simulink model "HvacCtrlrAirMFlowFrnt"  (R2022b)
settings: Solver=FixedStepDiscrete  StartTime=0.0  StopTime=10.0  FixedStep=0.2  RelTol=1e-3
(part 1 of 2)
settings Model: Creator=blue.ge  LastModifiedBy=blue.ge
settings DataIOCC: SaveFormat=StructureWithTime
settings OptimizationCC: DefaultParameterBehavior=Inlined  EnhancedBackFolding=on  PassReuseOutputArgsAs=Individual arguments  ZeroExternalMemoryAtStartup=off  ZeroInternalMemoryAtStartup=off  OptimizeBlockOrder=speed  DifferentSizesBufferReuse=on  OptimizationCustomize=off  EfficientTunableParamExpr=on
settings DebuggingCC: ParameterTunabilityLossMsg=error
settings ModelReferenceCC: ModelReferenceNumInstancesAllowed=Single
settings SFSimCC: MATLABDynamicMemAlloc=off
settings RTWCC: Description=AUTOSAR  SystemTargetFile=autosar.tlc  GenCodeOnly=on  TemplateMakefile=ert_default_tmf
settings CodeAppCC: ForceParamTrailComments=on  IgnoreCustomStorageClasses=off  OperatorAnnotations=on  SimulinkDataObjDesc=on  SFDataObjDesc=on  InsertBlockDesc=on
settings STFCustomTargetCC: TargetLangStandard=C99 (ISO)  MultiwordLength=256  ParMdlRefBuildCompliant=on  CompOptLevelCompliant=on  IncludeMdlTerminateFcn=off  CombineOutputUpdateFcns=on  SuppressErrorStatus=on  ERTCustomFileBanners=on  SupportNonFinite=off  SupportComplex=off  SupportContinuousTime=off  SupportNonInlinedSFcns=off  RemoveResetFunc=on  MATLABClassNameForMDSCustomization=Simulink.SoftwareTarget.ERTCustomization  AutosarCompliant=on  UseToolchainInfoCompliant=on  LookupTableObjectStructAxisOrder=2,1,3,4,...  LUTObjectStructOrderEvenSpacing=Size,Table,Breakpoints  SystemTargetFile=autosar.tlc
settings ERTTargetCC: TargetLangStandard=C99 (ISO)  ConcurrentExecutionCompliant=off  IncludeMdlTerminateFcn=off  SuppressErrorStatus=on  SupportNonFinite=off  SupportComplex=off  ModelStepFunctionPrototypeControlCompliant=off  CPPClassGenCompliant=off  AutosarCompliant=on  LookupTableObjectStructAxisOrder=2,1,3,4,...  LUTObjectStructOrderEvenSpacing=Size,Table,Breakpoints  InlinedParameterPlacement=NonHierarchical  ERTCustomFileTemplate=example_file_process.tlc

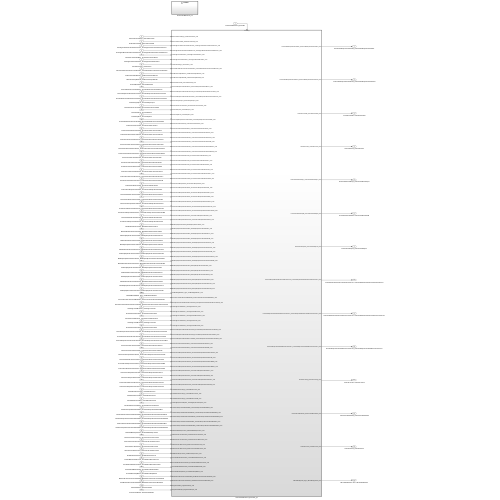
[thumbnail: Simulink's stored preview of the top-level diagram]
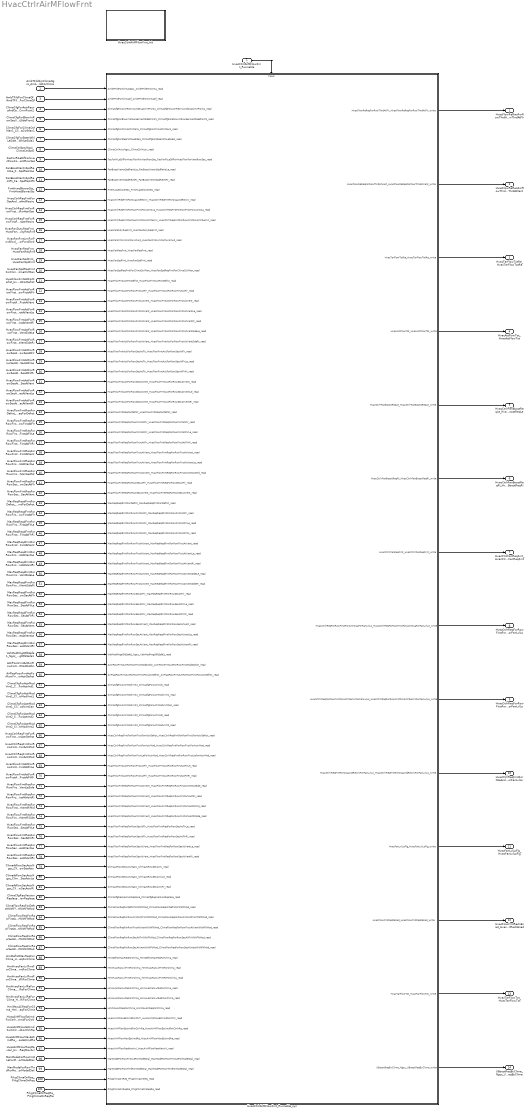
== system HvacCtrlrAirMFlowFrnt ==
  HvacCtrlrAirMFlowFrnt_Runnable : Inport  SampleTime=0.2  OutputFunctionCall=on
  AmbTFildForClimaNgcc_AmbTFildForClima : Inport  Port=2  OutDataTypeStr=T30  PortDimensions=1
  AmbTFildForClimaQf_AmbTFildForClimaQf : Inport  Port=3  OutDataTypeStr=Enum: GenQf1  PortDimensions=1
  ClimaCfgForActrRecircAndOsaCmnPrsnt1_ClimaCfgForActrRecircAndOsaCmnPrsnt1 : Inport  Port=4  OutDataTypeStr=Enum: ClimaCfgForActrRecircAndOsaCmnPrsnt1  PortDimensions=1
  ClimaCfgForBlowrInRowSecVentSidePrsnt1_ClimaCfgForBlowrInRowSecVentSidePrsnt1 : Inport  Port=5  OutDataTypeStr=Boolean  PortDimensions=1
  ClimaCfgForClimaCtrlMan1_ClimaCfgForClimaCtrlMan1 : Inport  Port=6  OutDataTypeStr=Boolean  PortDimensions=1
  ClimaCfgForSteerWhlLeSide1_ClimaCfgForSteerWhlLeSide1 : Inport  Port=7  OutDataTypeStr=Boolean  PortDimensions=1
  ClimaCtrlActvNgcc_ClimaCtrlActv : Inport  Port=8  OutDataTypeStr=Boolean  PortDimensions=1
  FacForRiLeDifForHvacFlowForVentRowSec_FacForRiLeDifForHvacFlowForVentRowSec : Inport  Port=9  OutDataTypeStr=Fac30  PortDimensions=1
  FanBoostVentnSpdReIntLe_FanBoostVentnSpdReIntLe : Inport  Port=10  OutDataTypeStr=HvacFanSpd  PortDimensions=1
  FanBoostVentnSpdReIntRi_FanBoostVentnSpdReIntRi : Inport  Port=11  OutDataTypeStr=HvacFanSpd  PortDimensions=1
  FrntHvacBlowerSts_FrntHvacBlowerSts : Inport  Port=12  OutDataTypeStr=Enum: HvacFanSts  PortDimensions=1
  HvacCtrlrReqFrntForOsaAndRecirc_HvacCtrlrReqFrntForOsaAndRecirc : Inport  Port=13  OutDataTypeStr=Perc30  PortDimensions=1
  HvacCtrlrReqFrntForRowFirstForActrOsa_HvacCtrlrReqFrntForRowFirstForActrOsa : Inport  Port=14  OutDataTypeStr=Perc30  PortDimensions=1
  HvacCtrlrReqFrntForRowFirstForActrRecirc_HvacCtrlrReqFrntForRowFirstForActrRecirc : Inport  Port=15  OutDataTypeStr=Perc30  PortDimensions=1
  HvacFanDutyReqFrnt_HvacFanDutyReqFrnt : Inport  Port=16  OutMin=0  OutMax=100  OutDataTypeStr=Perc9  PortDimensions=1
  HvacFanFrntLimForPwrAllwd_HvacFanFrntLimForPwrAllwd : Inport  Port=17  OutDataTypeStr=Enum: HvacFanFrntLimForPwrAllwd  PortDimensions=1
  HvacFanReqFrnt_HvacFanReqFrnt : Inport  Port=18  OutDataTypeStr=HvacFanSpd  PortDimensions=1
  HvacFanSpdFrnt_HvacFanSpdFrnt : Inport  Port=19  OutDataTypeStr=HvacFanSpd  PortDimensions=1
  HvacFanSpdReqFrntForClimaCtrlMan_HvacFanSpdReqFrntForClimaCtrlMan : Inport  Port=20  OutDataTypeStr=FanSpd1  PortDimensions=1
  HvacFlowFrntActForDefrst_HvacFlowFrntActForDefrst : Inport  Port=21  OutDataTypeStr=AirFlow30  PortDimensions=1
  HvacFlowFrntActForRowFirstAtFlr_HvacFlowFrntActForRowFirstAtFlr : Inport  Port=22  OutDataTypeStr=AirFlow30  PortDimensions=1
  HvacFlowFrntActForRowFirstAtVent_HvacFlowFrntActForRowFirstAtVent : Inport  Port=23  OutDataTypeStr=AirFlow30  PortDimensions=1
  HvacFlowFrntActForRowFirstAtVent_HvacFlowFrntActForRowFirstAtVentLe : Inport  Port=24  OutDataTypeStr=AirFlow30  PortDimensions=1
  HvacFlowFrntActForRowFirstAtVent_HvacFlowFrntActForRowFirstAtVentRi : Inport  Port=25  OutDataTypeStr=AirFlow30  PortDimensions=1
  HvacFlowFrntActForRowFirstAtVent_HvacFlowFrntActForRowFirstAtVentSideLe : Inport  Port=26  OutDataTypeStr=AirFlow30  PortDimensions=1
  HvacFlowFrntActForRowFirstAtVent_HvacFlowFrntActForRowFirstAtVentSideRi : Inport  Port=27  OutDataTypeStr=AirFlow30  PortDimensions=1
  HvacFlowFrntActForRowSecAtFlr_HvacFlowFrntActForRowSecAtFlr : Inport  Port=28  OutDataTypeStr=AirFlow30  PortDimensions=1
  HvacFlowFrntActForRowSecAtFlr_HvacFlowFrntActForRowSecAtFlrLe : Inport  Port=29  OutDataTypeStr=AirFlow30  PortDimensions=1
  HvacFlowFrntActForRowSecAtFlr_HvacFlowFrntActForRowSecAtFlrRi : Inport  Port=30  OutDataTypeStr=AirFlow30  PortDimensions=1
  HvacFlowFrntActForRowSecAtVent_HvacFlowFrntActForRowSecAtVent : Inport  Port=31  OutDataTypeStr=AirFlow30  PortDimensions=1
  HvacFlowFrntActForRowSecAtVent_HvacFlowFrntActForRowSecAtVentLe : Inport  Port=32  OutDataTypeStr=AirFlow30  PortDimensions=1
  HvacFlowFrntActForRowSecAtVent_HvacFlowFrntActForRowSecAtVentRi : Inport  Port=33  OutDataTypeStr=AirFlow30  PortDimensions=1
  HvacFlowFrntReqForDefrst_HvacFlowFrntReqForDefrst : Inport  Port=34  OutDataTypeStr=AirFlow30  PortDimensions=1
  HvacFlowFrntReqForRowFirstAtFlr_HvacFlowFrntReqForRowFirstAtFlr : Inport  Port=35  OutDataTypeStr=AirFlow30  PortDimensions=1
  HvacFlowFrntReqForRowFirstAtFlr_HvacFlowFrntReqForRowFirstAtFlrLe : Inport  Port=36  OutDataTypeStr=AirFlow30  PortDimensions=1
  HvacFlowFrntReqForRowFirstAtFlr_HvacFlowFrntReqForRowFirstAtFlrRi : Inport  Port=37  OutDataTypeStr=AirFlow30  PortDimensions=1
  HvacFlowFrntReqForRowFirstAtVent_HvacFlowFrntReqForRowFirstAtVent : Inport  Port=38  OutDataTypeStr=AirFlow30  PortDimensions=1
  HvacFlowFrntReqForRowFirstAtVent_HvacFlowFrntReqForRowFirstAtVentLe : Inport  Port=39  OutDataTypeStr=AirFlow30  PortDimensions=1
  HvacFlowFrntReqForRowFirstAtVent_HvacFlowFrntReqForRowFirstAtVentLeMid : Inport  Port=40  OutDataTypeStr=AirFlow30  PortDimensions=1
  HvacFlowFrntReqForRowSecAtFlr_HvacFlowFrntReqForRowSecAtFlr : Inport  Port=41  OutDataTypeStr=AirFlow30  PortDimensions=1
  HvacFlowFrntReqForRowSecAtVent_HvacFlowFrntReqForRowSecAtVent : Inport  Port=42  OutDataTypeStr=AirFlow30  PortDimensions=1
  MaxReqReqdFrntForDefrst_MaxReqReqdFrntForDefrst : Inport  Port=43  OutDataTypeStr=Boolean  PortDimensions=1
  MaxReqReqdFrntForRowFirstAtFlr_MaxReqReqdFrntForRowFirstAtFlr : Inport  Port=44  OutDataTypeStr=Boolean  PortDimensions=1
  MaxReqReqdFrntForRowFirstAtFlr_MaxReqReqdFrntForRowFirstAtFlrLe : Inport  Port=45  OutDataTypeStr=Boolean  PortDimensions=1
  MaxReqReqdFrntForRowFirstAtFlr_MaxReqReqdFrntForRowFirstAtFlrRi : Inport  Port=46  OutDataTypeStr=Boolean  PortDimensions=1
  MaxReqReqdFrntForRowFirstAtVent_MaxReqReqdFrntForRowFirstAtVent : Inport  Port=47  OutDataTypeStr=Boolean  PortDimensions=1
  MaxReqReqdFrntForRowFirstAtVent_MaxReqReqdFrntForRowFirstAtVentLe : Inport  Port=48  OutDataTypeStr=Boolean  PortDimensions=1
  MaxReqReqdFrntForRowFirstAtVent_MaxReqReqdFrntForRowFirstAtVentRi : Inport  Port=49  OutDataTypeStr=Boolean  PortDimensions=1
  MaxReqReqdFrntForRowFirstAtVent_MaxReqReqdFrntForRowFirstAtVentSideLe : Inport  Port=50  OutDataTypeStr=Boolean  PortDimensions=1
  MaxReqReqdFrntForRowFirstAtVent_MaxReqReqdFrntForRowFirstAtVentSideRi : Inport  Port=51  OutDataTypeStr=Boolean  PortDimensions=1
  MaxReqReqdFrntForRowSecAtFlr_MaxReqReqdFrntForRowSecAtFlr : Inport  Port=52  OutDataTypeStr=Boolean  PortDimensions=1
  MaxReqReqdFrntForRowSecAtFlr_MaxReqReqdFrntForRowSecAtFlrLe : Inport  Port=53  OutDataTypeStr=Boolean  PortDimensions=1
  MaxReqReqdFrntForRowSecAtFlr_MaxReqReqdFrntForRowSecAtFlrRi : Inport  Port=54  OutDataTypeStr=Boolean  PortDimensions=1
  MaxReqReqdFrntForRowSecAtVent_MaxReqReqdFrntForRowSecAtVent : Inport  Port=55  OutDataTypeStr=Boolean  PortDimensions=1
  MaxReqReqdFrntForRowSecAtVent_MaxReqReqdFrntForRowSecAtVentLe : Inport  Port=56  OutDataTypeStr=Boolean  PortDimensions=1
  MaxReqReqdFrntForRowSecAtVent_MaxReqReqdFrntForRowSecAtVentRi : Inport  Port=57  OutDataTypeStr=Boolean  PortDimensions=1
  VehModMngtGlbSafe1_Ngcc_VehModMngtGlbSafe1 : Inport  Port=58  OutDataTypeStr=Bus: VehModMngtGlbSafe1  PortDimensions=1  BusOutputAsStruct=on
  ActrPosnFrntActForRowFirstModDistbn_ActrPosnFrntActForRowFirstModDistbn : Inport  Port=59  OutMin=0  OutMax=100  OutDataTypeStr=Perc9  PortDimensions=1
  AirFlapPosnFrntActForRowFirstForActrDefrst_AirFlapPosnFrntActForRowFirstForActrDefrst : Inport  Port=60  OutMin=0  OutMax=100  OutDataTypeStr=Perc9  PortDimensions=1
  ClimaCfgForActrModVrnt1_ClimaCfgForActrInd1 : Inport  Port=61  OutDataTypeStr=Enum: ClimaCfgForActrInd1  PortDimensions=1
  ClimaCfgForActrModVrnt1_ClimaCfgForActrModVrnt1 : Inport  Port=62  OutDataTypeStr=Enum: ClimaCfgForActrModVrnt1  PortDimensions=1
  ClimaCfgForActrModVrnt1_ClimaCfgForActrModVrntCen : Inport  Port=63  OutDataTypeStr=Enum: ClimaCfgForActrModVrntCen  PortDimensions=1
  ClimaCfgForActrModVrnt2_ClimaCfgForActrInd2 : Inport  Port=64  OutDataTypeStr=Enum: ClimaCfgForActrInd2  PortDimensions=1
  ClimaCfgForActrModVrnt2_ClimaCfgForActrModVrnt2 : Inport  Port=65  OutDataTypeStr=Enum: ClimaCfgForActrModVrnt2  PortDimensions=1
  HvacCtrlrReqFrntForRowFirstForActrDefrst_HvacCtrlrReqFrntForRowFirstForActrDefrst : Inport  Port=66  OutDataTypeStr=Perc30  PortDimensions=1
  HvacCtrlrReqFrntForRowFirstForActrMod_HvacCtrlrReqFrntForRowFirstForActrMod : Inport  Port=67  OutDataTypeStr=Perc30  PortDimensions=1
  HvacCtrlrReqFrntForRowFirstLeForActrMod_HvacCtrlrReqFrntForRowFirstLeForActrMod : Inport  Port=68  OutDataTypeStr=Perc30  PortDimensions=1
  HvacFlowFrntActForRowFirstAtFlr_HvacFlowFrntActForRowFirstAtFlrLe : Inport  Port=69  OutDataTypeStr=AirFlow30  PortDimensions=1
  HvacFlowFrntActForRowFirstAtFlr_HvacFlowFrntActForRowFirstAtFlrRi : Inport  Port=70  OutDataTypeStr=AirFlow30  PortDimensions=1
  HvacFlowFrntReqForRowFirstAtVent_HvacFlowFrntReqForRowFirstAtVentLeSide : Inport  Port=71  OutDataTypeStr=AirFlow30  PortDimensions=1
  HvacFlowFrntReqForRowFirstAtVent_HvacFlowFrntReqForRowFirstAtVentRi : Inport  Port=72  OutDataTypeStr=AirFlow30  PortDimensions=1
  HvacFlowFrntReqForRowFirstAtVent_HvacFlowFrntReqForRowFirstAtVentRiMid : Inport  Port=73  OutDataTypeStr=AirFlow30  PortDimensions=1
  HvacFlowFrntReqForRowFirstAtVent_HvacFlowFrntReqForRowFirstAtVentRiSide : Inport  Port=74  OutDataTypeStr=AirFlow30  PortDimensions=1
  HvacFlowFrntReqForRowSecAtFlr_HvacFlowFrntReqForRowSecAtFlrLe : Inport  Port=75  OutDataTypeStr=AirFlow30  PortDimensions=1
  HvacFlowFrntReqForRowSecAtFlr_HvacFlowFrntReqForRowSecAtFlrRi : Inport  Port=76  OutDataTypeStr=AirFlow30  PortDimensions=1
  HvacFlowFrntReqForRowSecAtVent_HvacFlowFrntReqForRowSecAtVentLe : Inport  Port=77  OutDataTypeStr=AirFlow30  PortDimensions=1
  HvacFlowFrntReqForRowSecAtVent_HvacFlowFrntReqForRowSecAtVentRi : Inport  Port=78  OutDataTypeStr=AirFlow30  PortDimensions=1
  ClimaAtRowSecActvNgcc_ClimaAtRowSecActv : Inport  Port=79  OutDataTypeStr=Boolean  PortDimensions=1
  ClimaAtRowSecActvNgcc_ClimaAtRowSecActvLe : Inport  Port=80  OutDataTypeStr=Boolean  PortDimensions=1
  ClimaAtRowSecActvNgcc_ClimaAtRowSecActvRi : Inport  Port=81  OutDataTypeStr=Boolean  PortDimensions=1
  ClimaCfgReqVersionReplace_ClimaCfgReqVersionReplace : Inport  Port=82  OutDataTypeStr=UInt8  PortDimensions=1
  ClimaFlowReqForDefrstWithFltMod_ClimaFlowReqForDefrstWithFltMod : Inport  Port=83  OutDataTypeStr=AirFlow30  PortDimensions=1
  ClimaFlowReqForRowFirstAtFlrWithFltMod_ClimaFlowReqForRowFirstAtFlrWithFltMod : Inport  Port=84  OutDataTypeStr=AirFlow30  PortDimensions=1
  ClimaFlowReqForRowFirstAtVentWithFltMod_ClimaFlowReqForRowFirstAtVentWithFltMod : Inport  Port=85  OutDataTypeStr=AirFlow30  PortDimensions=1
  ClimaFlowReqForRowSecAtFlrWithFltMod_ClimaFlowReqForRowSecAtFlrWithFltMod : Inport  Port=86  OutDataTypeStr=AirFlow30  PortDimensions=1
  ClimaFlowReqForRowSecAtVentWithFltMod_ClimaFlowReqForRowSecAtVentWithFltMod : Inport  Port=87  OutDataTypeStr=AirFlow30  PortDimensions=1
  HmiDefrstMaxReqForClima_HmiDefrstMaxReqForClima : Inport  Port=88  OutDataTypeStr=Enum: ActrReq  PortDimensions=1
  HmiHvacFanLvlFrntForClima_HmiHvacFanLvlFrntForClima : Inport  Port=89  OutDataTypeStr=Enum: HmiHvacFanLvl  PortDimensions=1
  HmiHvacFanLvlFrntForClima_HmiHvacFanLvlFrntRiForClima : Inport  Port=90  OutDataTypeStr=Enum: HmiHvacFanLvl  PortDimensions=1
  HmiHvacFanLvlReForClima_HmiHvacFanLvlReForClima : Inport  Port=91  OutDataTypeStr=Enum: HmiHvacFanLvl  PortDimensions=1
  HmiHvacFanLvlReForClima_HmiHvacFanLvlReRiForClima : Inport  Port=92  OutDataTypeStr=Enum: HmiHvacFanLvl  PortDimensions=1
  HmiMaxACReqForClima_HmiMaxACReqForClima : Inport  Port=93  OutDataTypeStr=Enum: OnOff1  PortDimensions=1
  HvacAirMFlowEstimdForCtrlr_HvacAirMFlowEstimdForCtrlr : Inport  Port=94  OutDataTypeStr=AirFlow30  PortDimensions=1
  HvacAirMFlowEstimdForCtrlrRe_HvacAirMFlowEstimdForCtrlrRe : Inport  Port=95  OutDataTypeStr=AirFlow30  PortDimensions=1
  HvacAirMFlowMaxEstimdRe_HvacAirMFlowMaxEstimdRe : Inport  Port=96  OutDataTypeStr=AirFlow30  PortDimensions=1
  HvacAirMFlowReqMaxAvl_HvacAirMFlowReqMaxAvl : Inport  Port=97  OutDataTypeStr=AirFlow30  PortDimensions=1
  ManModeForRowFirstLeForModelDial_ManModeForRowFirstLeForModelDial : Inport  Port=98  OutDataTypeStr=Enum: HmiCmptmtAirDistbnFrnt  PortDimensions=1
  ManModeForRowThrdForModelDial_ManModeForRowThrdForModelDial : Inport  Port=99  OutDataTypeStr=Enum: HmiCmptmtAirDistbnFrnt  PortDimensions=1
  PrkgClimaOnReq_PrkgClimaOnReq : Inport  Port=100  OutDataTypeStr=Boolean  PortDimensions=1
  PrkgClimaOnReqRe_PrkgClimaOnReqRe : Inport  Port=101  OutDataTypeStr=Enum: OnOff1  PortDimensions=1
  HvacCtrlrAirMFlowFrnt_Init : Subsystem
  HvacCtrlrAirMFlowFrnt_Runnable_sys : Subsystem
  HvacFlowReReqForRowThrdAtFlr_HvacFlowReReqForRowThrdAtFlr : Outport  OutDataTypeStr=AirFlow30  PortDimensions=1
  HvacFlowReReqForRowThrdAtVent_HvacFlowReReqForRowThrdAtVent : Outport  Port=2  OutDataTypeStr=AirFlow30  PortDimensions=1
  HvacTarFlowTotRe_HvacTarFlowTotRe : Outport  Port=3  OutDataTypeStr=AirFlow30  PortDimensions=1
  HvacActFlowTot_HvacActFlowTot : Outport  Port=4  OutDataTypeStr=AirFlow30  PortDimensions=1
  HvacCtrlrFanBoostReqLe_HvacCtrlrFanBoostReqLe : Outport  Port=5  OutDataTypeStr=Perc30  PortDimensions=1
  HvacCtrlrFanBoostReqRi_HvacCtrlrFanBoostReqRi : Outport  Port=6  OutDataTypeStr=Perc30  PortDimensions=1
  HvacCtrlrFanReqFrnt_HvacCtrlrFanReqFrnt : Outport  Port=7  OutDataTypeStr=FanSpd1  PortDimensions=1
  HvacCtrlrReqForRowFirstForActrOsaForFanLvlLo_HvacCtrlrReqForRowFirstForActrOsaForFanLvlLo : Outport  Port=8  OutDataTypeStr=Perc30  PortDimensions=1
  HvacCtrlrReqForRowFirstForActrRecircForFanLvlLo_HvacCtrlrReqForRowFirstForActrRecircForFanLvlLo : Outport  Port=9  OutDataTypeStr=Perc30  PortDimensions=1
  HvacCtrlrReqFrntForOsaAndRecircForFanLvlLo_HvacCtrlrReqFrntForOsaAndRecircForFanLvlLo : Outport  Port=10  OutDataTypeStr=Perc30  PortDimensions=1
  HvacFanLvlLoFlg_HvacFanLvlLoFlg : Outport  Port=11  OutDataTypeStr=Boolean  PortDimensions=1
  HvacFlowFrntRednEnad_HvacFlowFrntRednEnad : Outport  Port=12  OutDataTypeStr=Boolean  PortDimensions=1
  HvacTarFlowTot_HvacTarFlowTot : Outport  Port=13  OutDataTypeStr=AirFlow30  PortDimensions=1
  UBoostReqByClima_Ngcc_UBoostReqByClima : Outport  Port=14  OutDataTypeStr=Enum: ReqSts1  PortDimensions=1
  wires:
  AmbTFildForClimaNgcc_AmbTFildForClima/1 -> HvacCtrlrAirMFlowFrnt_Runnable_sys/1(AmbTFildForClimaNgcc_AmbTFildForClima_read)
  AmbTFildForClimaQf_AmbTFildForClimaQf/1 -> HvacCtrlrAirMFlowFrnt_Runnable_sys/2(AmbTFildForClimaQf_AmbTFildForClimaQf_read)
  ClimaCfgForActrRecircAndOsaCmnPrsnt1_ClimaCfgForActrRecircAndOsaCmnPrsnt1/1 -> HvacCtrlrAirMFlowFrnt_Runnable_sys/3(ClimaCfgForActrRecircAndOsaCmnPrsnt1_ClimaCfgForActrRecircAndOsaCmnPrsnt1_read)
  ClimaCfgForBlowrInRowSecVentSidePrsnt1_ClimaCfgForBlowrInRowSecVentSidePrsnt1/1 -> HvacCtrlrAirMFlowFrnt_Runnable_sys/4(ClimaCfgForBlowrInRowSecVentSidePrsnt1_ClimaCfgForBlowrInRowSecVentSidePrsnt1_read)
  ClimaCfgForClimaCtrlMan1_ClimaCfgForClimaCtrlMan1/1 -> HvacCtrlrAirMFlowFrnt_Runnable_sys/5(ClimaCfgForClimaCtrlMan1_ClimaCfgForClimaCtrlMan1_read)
  ClimaCfgForSteerWhlLeSide1_ClimaCfgForSteerWhlLeSide1/1 -> HvacCtrlrAirMFlowFrnt_Runnable_sys/6(ClimaCfgForSteerWhlLeSide1_ClimaCfgForSteerWhlLeSide1_read)
  ClimaCtrlActvNgcc_ClimaCtrlActv/1 -> HvacCtrlrAirMFlowFrnt_Runnable_sys/7(ClimaCtrlActvNgcc_ClimaCtrlActv_read)
  FacForRiLeDifForHvacFlowForVentRowSec_FacForRiLeDifForHvacFlowForVentRowSec/1 -> HvacCtrlrAirMFlowFrnt_Runnable_sys/8(FacForRiLeDifForHvacFlowForVentRowSec_FacForRiLeDifForHvacFlowForVentRowSec_read)
  FanBoostVentnSpdReIntLe_FanBoostVentnSpdReIntLe/1 -> HvacCtrlrAirMFlowFrnt_Runnable_sys/9(FanBoostVentnSpdReIntLe_FanBoostVentnSpdReIntLe_read)
  FanBoostVentnSpdReIntRi_FanBoostVentnSpdReIntRi/1 -> HvacCtrlrAirMFlowFrnt_Runnable_sys/10(FanBoostVentnSpdReIntRi_FanBoostVentnSpdReIntRi_read)
  FrntHvacBlowerSts_FrntHvacBlowerSts/1 -> HvacCtrlrAirMFlowFrnt_Runnable_sys/11(FrntHvacBlowerSts_FrntHvacBlowerSts_read)
  HvacCtrlrReqFrntForOsaAndRecirc_HvacCtrlrReqFrntForOsaAndRecirc/1 -> HvacCtrlrAirMFlowFrnt_Runnable_sys/12(HvacCtrlrReqFrntForOsaAndRecirc_HvacCtrlrReqFrntForOsaAndRecirc_read)
  HvacCtrlrReqFrntForRowFirstForActrOsa_HvacCtrlrReqFrntForRowFirstForActrOsa/1 -> HvacCtrlrAirMFlowFrnt_Runnable_sys/13(HvacCtrlrReqFrntForRowFirstForActrOsa_HvacCtrlrReqFrntForRowFirstForActrOsa_read)
  HvacCtrlrReqFrntForRowFirstForActrRecirc_HvacCtrlrReqFrntForRowFirstForActrRecirc/1 -> HvacCtrlrAirMFlowFrnt_Runnable_sys/14(HvacCtrlrReqFrntForRowFirstForActrRecirc_HvacCtrlrReqFrntForRowFirstForActrRecirc_read)
  HvacFanDutyReqFrnt_HvacFanDutyReqFrnt/1 -> HvacCtrlrAirMFlowFrnt_Runnable_sys/15(HvacFanDutyReqFrnt_HvacFanDutyReqFrnt_read)
  HvacFanFrntLimForPwrAllwd_HvacFanFrntLimForPwrAllwd/1 -> HvacCtrlrAirMFlowFrnt_Runnable_sys/16(HvacFanFrntLimForPwrAllwd_HvacFanFrntLimForPwrAllwd_read)
  HvacFanReqFrnt_HvacFanReqFrnt/1 -> HvacCtrlrAirMFlowFrnt_Runnable_sys/17(HvacFanReqFrnt_HvacFanReqFrnt_read)
  HvacFanSpdFrnt_HvacFanSpdFrnt/1 -> HvacCtrlrAirMFlowFrnt_Runnable_sys/18(HvacFanSpdFrnt_HvacFanSpdFrnt_read)
  HvacFanSpdReqFrntForClimaCtrlMan_HvacFanSpdReqFrntForClimaCtrlMan/1 -> HvacCtrlrAirMFlowFrnt_Runnable_sys/19(HvacFanSpdReqFrntForClimaCtrlMan_HvacFanSpdReqFrntForClimaCtrlMan_read)
  HvacFlowFrntActForDefrst_HvacFlowFrntActForDefrst/1 -> HvacCtrlrAirMFlowFrnt_Runnable_sys/20(HvacFlowFrntActForDefrst_HvacFlowFrntActForDefrst_read)
  HvacFlowFrntActForRowFirstAtFlr_HvacFlowFrntActForRowFirstAtFlr/1 -> HvacCtrlrAirMFlowFrnt_Runnable_sys/21(HvacFlowFrntActForRowFirstAtFlr_HvacFlowFrntActForRowFirstAtFlr_read)
  HvacFlowFrntActForRowFirstAtVent_HvacFlowFrntActForRowFirstAtVent/1 -> HvacCtrlrAirMFlowFrnt_Runnable_sys/22(HvacFlowFrntActForRowFirstAtVent_HvacFlowFrntActForRowFirstAtVent_read)
  HvacFlowFrntActForRowFirstAtVent_HvacFlowFrntActForRowFirstAtVentLe/1 -> HvacCtrlrAirMFlowFrnt_Runnable_sys/23(HvacFlowFrntActForRowFirstAtVent_HvacFlowFrntActForRowFirstAtVentLe_read)
  HvacFlowFrntActForRowFirstAtVent_HvacFlowFrntActForRowFirstAtVentRi/1 -> HvacCtrlrAirMFlowFrnt_Runnable_sys/24(HvacFlowFrntActForRowFirstAtVent_HvacFlowFrntActForRowFirstAtVentRi_read)
  HvacFlowFrntActForRowFirstAtVent_HvacFlowFrntActForRowFirstAtVentSideLe/1 -> HvacCtrlrAirMFlowFrnt_Runnable_sys/25(HvacFlowFrntActForRowFirstAtVent_HvacFlowFrntActForRowFirstAtVentSideLe_read)
  HvacFlowFrntActForRowFirstAtVent_HvacFlowFrntActForRowFirstAtVentSideRi/1 -> HvacCtrlrAirMFlowFrnt_Runnable_sys/26(HvacFlowFrntActForRowFirstAtVent_HvacFlowFrntActForRowFirstAtVentSideRi_read)
  HvacFlowFrntActForRowSecAtFlr_HvacFlowFrntActForRowSecAtFlr/1 -> HvacCtrlrAirMFlowFrnt_Runnable_sys/27(HvacFlowFrntActForRowSecAtFlr_HvacFlowFrntActForRowSecAtFlr_read)
  HvacFlowFrntActForRowSecAtFlr_HvacFlowFrntActForRowSecAtFlrLe/1 -> HvacCtrlrAirMFlowFrnt_Runnable_sys/28(HvacFlowFrntActForRowSecAtFlr_HvacFlowFrntActForRowSecAtFlrLe_read)
  HvacFlowFrntActForRowSecAtFlr_HvacFlowFrntActForRowSecAtFlrRi/1 -> HvacCtrlrAirMFlowFrnt_Runnable_sys/29(HvacFlowFrntActForRowSecAtFlr_HvacFlowFrntActForRowSecAtFlrRi_read)
  HvacFlowFrntActForRowSecAtVent_HvacFlowFrntActForRowSecAtVent/1 -> HvacCtrlrAirMFlowFrnt_Runnable_sys/30(HvacFlowFrntActForRowSecAtVent_HvacFlowFrntActForRowSecAtVent_read)
  HvacFlowFrntActForRowSecAtVent_HvacFlowFrntActForRowSecAtVentLe/1 -> HvacCtrlrAirMFlowFrnt_Runnable_sys/31(HvacFlowFrntActForRowSecAtVent_HvacFlowFrntActForRowSecAtVentLe_read)
  HvacFlowFrntActForRowSecAtVent_HvacFlowFrntActForRowSecAtVentRi/1 -> HvacCtrlrAirMFlowFrnt_Runnable_sys/32(HvacFlowFrntActForRowSecAtVent_HvacFlowFrntActForRowSecAtVentRi_read)
  HvacFlowFrntReqForDefrst_HvacFlowFrntReqForDefrst/1 -> HvacCtrlrAirMFlowFrnt_Runnable_sys/33(HvacFlowFrntReqForDefrst_HvacFlowFrntReqForDefrst_read)
  HvacFlowFrntReqForRowFirstAtFlr_HvacFlowFrntReqForRowFirstAtFlr/1 -> HvacCtrlrAirMFlowFrnt_Runnable_sys/34(HvacFlowFrntReqForRowFirstAtFlr_HvacFlowFrntReqForRowFirstAtFlr_read)
  HvacFlowFrntReqForRowFirstAtFlr_HvacFlowFrntReqForRowFirstAtFlrLe/1 -> HvacCtrlrAirMFlowFrnt_Runnable_sys/35(HvacFlowFrntReqForRowFirstAtFlr_HvacFlowFrntReqForRowFirstAtFlrLe_read)
  HvacFlowFrntReqForRowFirstAtFlr_HvacFlowFrntReqForRowFirstAtFlrRi/1 -> HvacCtrlrAirMFlowFrnt_Runnable_sys/36(HvacFlowFrntReqForRowFirstAtFlr_HvacFlowFrntReqForRowFirstAtFlrRi_read)
  HvacFlowFrntReqForRowFirstAtVent_HvacFlowFrntReqForRowFirstAtVent/1 -> HvacCtrlrAirMFlowFrnt_Runnable_sys/37(HvacFlowFrntReqForRowFirstAtVent_HvacFlowFrntReqForRowFirstAtVent_read)
  HvacFlowFrntReqForRowFirstAtVent_HvacFlowFrntReqForRowFirstAtVentLe/1 -> HvacCtrlrAirMFlowFrnt_Runnable_sys/38(HvacFlowFrntReqForRowFirstAtVent_HvacFlowFrntReqForRowFirstAtVentLe_read)
  HvacFlowFrntReqForRowFirstAtVent_HvacFlowFrntReqForRowFirstAtVentLeMid/1 -> HvacCtrlrAirMFlowFrnt_Runnable_sys/39(HvacFlowFrntReqForRowFirstAtVent_HvacFlowFrntReqForRowFirstAtVentLeMid_read)
  HvacFlowFrntReqForRowSecAtFlr_HvacFlowFrntReqForRowSecAtFlr/1 -> HvacCtrlrAirMFlowFrnt_Runnable_sys/40(HvacFlowFrntReqForRowSecAtFlr_HvacFlowFrntReqForRowSecAtFlr_read)
  HvacFlowFrntReqForRowSecAtVent_HvacFlowFrntReqForRowSecAtVent/1 -> HvacCtrlrAirMFlowFrnt_Runnable_sys/41(HvacFlowFrntReqForRowSecAtVent_HvacFlowFrntReqForRowSecAtVent_read)
  MaxReqReqdFrntForDefrst_MaxReqReqdFrntForDefrst/1 -> HvacCtrlrAirMFlowFrnt_Runnable_sys/42(MaxReqReqdFrntForDefrst_MaxReqReqdFrntForDefrst_read)
  MaxReqReqdFrntForRowFirstAtFlr_MaxReqReqdFrntForRowFirstAtFlr/1 -> HvacCtrlrAirMFlowFrnt_Runnable_sys/43(MaxReqReqdFrntForRowFirstAtFlr_MaxReqReqdFrntForRowFirstAtFlr_read)
  MaxReqReqdFrntForRowFirstAtFlr_MaxReqReqdFrntForRowFirstAtFlrLe/1 -> HvacCtrlrAirMFlowFrnt_Runnable_sys/44(MaxReqReqdFrntForRowFirstAtFlr_MaxReqReqdFrntForRowFirstAtFlrLe_read)
  MaxReqReqdFrntForRowFirstAtFlr_MaxReqReqdFrntForRowFirstAtFlrRi/1 -> HvacCtrlrAirMFlowFrnt_Runnable_sys/45(MaxReqReqdFrntForRowFirstAtFlr_MaxReqReqdFrntForRowFirstAtFlrRi_read)
  MaxReqReqdFrntForRowFirstAtVent_MaxReqReqdFrntForRowFirstAtVent/1 -> HvacCtrlrAirMFlowFrnt_Runnable_sys/46(MaxReqReqdFrntForRowFirstAtVent_MaxReqReqdFrntForRowFirstAtVent_read)
  MaxReqReqdFrntForRowFirstAtVent_MaxReqReqdFrntForRowFirstAtVentLe/1 -> HvacCtrlrAirMFlowFrnt_Runnable_sys/47(MaxReqReqdFrntForRowFirstAtVent_MaxReqReqdFrntForRowFirstAtVentLe_read)
  MaxReqReqdFrntForRowFirstAtVent_MaxReqReqdFrntForRowFirstAtVentRi/1 -> HvacCtrlrAirMFlowFrnt_Runnable_sys/48(MaxReqReqdFrntForRowFirstAtVent_MaxReqReqdFrntForRowFirstAtVentRi_read)
  MaxReqReqdFrntForRowFirstAtVent_MaxReqReqdFrntForRowFirstAtVentSideLe/1 -> HvacCtrlrAirMFlowFrnt_Runnable_sys/49(MaxReqReqdFrntForRowFirstAtVent_MaxReqReqdFrntForRowFirstAtVentSideLe_read)
  MaxReqReqdFrntForRowFirstAtVent_MaxReqReqdFrntForRowFirstAtVentSideRi/1 -> HvacCtrlrAirMFlowFrnt_Runnable_sys/50(MaxReqReqdFrntForRowFirstAtVent_MaxReqReqdFrntForRowFirstAtVentSideRi_read)
  MaxReqReqdFrntForRowSecAtFlr_MaxReqReqdFrntForRowSecAtFlr/1 -> HvacCtrlrAirMFlowFrnt_Runnable_sys/51(MaxReqReqdFrntForRowSecAtFlr_MaxReqReqdFrntForRowSecAtFlr_read)
  MaxReqReqdFrntForRowSecAtFlr_MaxReqReqdFrntForRowSecAtFlrLe/1 -> HvacCtrlrAirMFlowFrnt_Runnable_sys/52(MaxReqReqdFrntForRowSecAtFlr_MaxReqReqdFrntForRowSecAtFlrLe_read)
  MaxReqReqdFrntForRowSecAtFlr_MaxReqReqdFrntForRowSecAtFlrRi/1 -> HvacCtrlrAirMFlowFrnt_Runnable_sys/53(MaxReqReqdFrntForRowSecAtFlr_MaxReqReqdFrntForRowSecAtFlrRi_read)
  MaxReqReqdFrntForRowSecAtVent_MaxReqReqdFrntForRowSecAtVent/1 -> HvacCtrlrAirMFlowFrnt_Runnable_sys/54(MaxReqReqdFrntForRowSecAtVent_MaxReqReqdFrntForRowSecAtVent_read)
  MaxReqReqdFrntForRowSecAtVent_MaxReqReqdFrntForRowSecAtVentLe/1 -> HvacCtrlrAirMFlowFrnt_Runnable_sys/55(MaxReqReqdFrntForRowSecAtVent_MaxReqReqdFrntForRowSecAtVentLe_read)
  MaxReqReqdFrntForRowSecAtVent_MaxReqReqdFrntForRowSecAtVentRi/1 -> HvacCtrlrAirMFlowFrnt_Runnable_sys/56(MaxReqReqdFrntForRowSecAtVent_MaxReqReqdFrntForRowSecAtVentRi_read)
  VehModMngtGlbSafe1_Ngcc_VehModMngtGlbSafe1/1 -> HvacCtrlrAirMFlowFrnt_Runnable_sys/57(VehModMngtGlbSafe1_Ngcc_VehModMngtGlbSafe1_read)
  ActrPosnFrntActForRowFirstModDistbn_ActrPosnFrntActForRowFirstModDistbn/1 -> HvacCtrlrAirMFlowFrnt_Runnable_sys/58(ActrPosnFrntActForRowFirstModDistbn_ActrPosnFrntActForRowFirstModDistbn_read)
  AirFlapPosnFrntActForRowFirstForActrDefrst_AirFlapPosnFrntActForRowFirstForActrDefrst/1 -> HvacCtrlrAirMFlowFrnt_Runnable_sys/59(AirFlapPosnFrntActForRowFirstForActrDefrst_AirFlapPosnFrntActForRowFirstForActrDefrst_read)
  ClimaCfgForActrModVrnt1_ClimaCfgForActrInd1/1 -> HvacCtrlrAirMFlowFrnt_Runnable_sys/60(ClimaCfgForActrModVrnt1_ClimaCfgForActrInd1_read)
  ClimaCfgForActrModVrnt1_ClimaCfgForActrModVrnt1/1 -> HvacCtrlrAirMFlowFrnt_Runnable_sys/61(ClimaCfgForActrModVrnt1_ClimaCfgForActrModVrnt1_read)
  ClimaCfgForActrModVrnt1_ClimaCfgForActrModVrntCen/1 -> HvacCtrlrAirMFlowFrnt_Runnable_sys/62(ClimaCfgForActrModVrnt1_ClimaCfgForActrModVrntCen_read)
  ClimaCfgForActrModVrnt2_ClimaCfgForActrInd2/1 -> HvacCtrlrAirMFlowFrnt_Runnable_sys/63(ClimaCfgForActrModVrnt2_ClimaCfgForActrInd2_read)
  ClimaCfgForActrModVrnt2_ClimaCfgForActrModVrnt2/1 -> HvacCtrlrAirMFlowFrnt_Runnable_sys/64(ClimaCfgForActrModVrnt2_ClimaCfgForActrModVrnt2_read)
  HvacCtrlrReqFrntForRowFirstForActrDefrst_HvacCtrlrReqFrntForRowFirstForActrDefrst/1 -> HvacCtrlrAirMFlowFrnt_Runnable_sys/65(HvacCtrlrReqFrntForRowFirstForActrDefrst_HvacCtrlrReqFrntForRowFirstForActrDefrst_read)
  HvacCtrlrReqFrntForRowFirstForActrMod_HvacCtrlrReqFrntForRowFirstForActrMod/1 -> HvacCtrlrAirMFlowFrnt_Runnable_sys/66(HvacCtrlrReqFrntForRowFirstForActrMod_HvacCtrlrReqFrntForRowFirstForActrMod_read)
  HvacCtrlrReqFrntForRowFirstLeForActrMod_HvacCtrlrReqFrntForRowFirstLeForActrMod/1 -> HvacCtrlrAirMFlowFrnt_Runnable_sys/67(HvacCtrlrReqFrntForRowFirstLeForActrMod_HvacCtrlrReqFrntForRowFirstLeForActrMod_read)
  HvacFlowFrntActForRowFirstAtFlr_HvacFlowFrntActForRowFirstAtFlrLe/1 -> HvacCtrlrAirMFlowFrnt_Runnable_sys/68(HvacFlowFrntActForRowFirstAtFlr_HvacFlowFrntActForRowFirstAtFlrLe_read)
  HvacFlowFrntActForRowFirstAtFlr_HvacFlowFrntActForRowFirstAtFlrRi/1 -> HvacCtrlrAirMFlowFrnt_Runnable_sys/69(HvacFlowFrntActForRowFirstAtFlr_HvacFlowFrntActForRowFirstAtFlrRi_read)
  HvacFlowFrntReqForRowFirstAtVent_HvacFlowFrntReqForRowFirstAtVentLeSide/1 -> HvacCtrlrAirMFlowFrnt_Runnable_sys/70(HvacFlowFrntReqForRowFirstAtVent_HvacFlowFrntReqForRowFirstAtVentLeSide_read)
  HvacFlowFrntReqForRowFirstAtVent_HvacFlowFrntReqForRowFirstAtVentRi/1 -> HvacCtrlrAirMFlowFrnt_Runnable_sys/71(HvacFlowFrntReqForRowFirstAtVent_HvacFlowFrntReqForRowFirstAtVentRi_read)
  HvacFlowFrntReqForRowFirstAtVent_HvacFlowFrntReqForRowFirstAtVentRiMid/1 -> HvacCtrlrAirMFlowFrnt_Runnable_sys/72(HvacFlowFrntReqForRowFirstAtVent_HvacFlowFrntReqForRowFirstAtVentRiMid_read)
  HvacFlowFrntReqForRowFirstAtVent_HvacFlowFrntReqForRowFirstAtVentRiSide/1 -> HvacCtrlrAirMFlowFrnt_Runnable_sys/73(HvacFlowFrntReqForRowFirstAtVent_HvacFlowFrntReqForRowFirstAtVentRiSide_read)
  HvacFlowFrntReqForRowSecAtFlr_HvacFlowFrntReqForRowSecAtFlrLe/1 -> HvacCtrlrAirMFlowFrnt_Runnable_sys/74(HvacFlowFrntReqForRowSecAtFlr_HvacFlowFrntReqForRowSecAtFlrLe_read)
  HvacFlowFrntReqForRowSecAtFlr_HvacFlowFrntReqForRowSecAtFlrRi/1 -> HvacCtrlrAirMFlowFrnt_Runnable_sys/75(HvacFlowFrntReqForRowSecAtFlr_HvacFlowFrntReqForRowSecAtFlrRi_read)
  HvacFlowFrntReqForRowSecAtVent_HvacFlowFrntReqForRowSecAtVentLe/1 -> HvacCtrlrAirMFlowFrnt_Runnable_sys/76(HvacFlowFrntReqForRowSecAtVent_HvacFlowFrntReqForRowSecAtVentLe_read)
  HvacFlowFrntReqForRowSecAtVent_HvacFlowFrntReqForRowSecAtVentRi/1 -> HvacCtrlrAirMFlowFrnt_Runnable_sys/77(HvacFlowFrntReqForRowSecAtVent_HvacFlowFrntReqForRowSecAtVentRi_read)
  ClimaAtRowSecActvNgcc_ClimaAtRowSecActv/1 -> HvacCtrlrAirMFlowFrnt_Runnable_sys/78(ClimaAtRowSecActvNgcc_ClimaAtRowSecActv_read)
  ClimaAtRowSecActvNgcc_ClimaAtRowSecActvLe/1 -> HvacCtrlrAirMFlowFrnt_Runnable_sys/79(ClimaAtRowSecActvNgcc_ClimaAtRowSecActvLe_read)
  ClimaAtRowSecActvNgcc_ClimaAtRowSecActvRi/1 -> HvacCtrlrAirMFlowFrnt_Runnable_sys/80(ClimaAtRowSecActvNgcc_ClimaAtRowSecActvRi_read)
  ClimaCfgReqVersionReplace_ClimaCfgReqVersionReplace/1 -> HvacCtrlrAirMFlowFrnt_Runnable_sys/81(ClimaCfgReqVersionReplace_ClimaCfgReqVersionReplace_read)
  ClimaFlowReqForDefrstWithFltMod_ClimaFlowReqForDefrstWithFltMod/1 -> HvacCtrlrAirMFlowFrnt_Runnable_sys/82(ClimaFlowReqForDefrstWithFltMod_ClimaFlowReqForDefrstWithFltMod_read)
  ClimaFlowReqForRowFirstAtFlrWithFltMod_ClimaFlowReqForRowFirstAtFlrWithFltMod/1 -> HvacCtrlrAirMFlowFrnt_Runnable_sys/83(ClimaFlowReqForRowFirstAtFlrWithFltMod_ClimaFlowReqForRowFirstAtFlrWithFltMod_read)
  ClimaFlowReqForRowFirstAtVentWithFltMod_ClimaFlowReqForRowFirstAtVentWithFltMod/1 -> HvacCtrlrAirMFlowFrnt_Runnable_sys/84(ClimaFlowReqForRowFirstAtVentWithFltMod_ClimaFlowReqForRowFirstAtVentWithFltMod_read)
  ClimaFlowReqForRowSecAtFlrWithFltMod_ClimaFlowReqForRowSecAtFlrWithFltMod/1 -> HvacCtrlrAirMFlowFrnt_Runnable_sys/85(ClimaFlowReqForRowSecAtFlrWithFltMod_ClimaFlowReqForRowSecAtFlrWithFltMod_read)
  ClimaFlowReqForRowSecAtVentWithFltMod_ClimaFlowReqForRowSecAtVentWithFltMod/1 -> HvacCtrlrAirMFlowFrnt_Runnable_sys/86(ClimaFlowReqForRowSecAtVentWithFltMod_ClimaFlowReqForRowSecAtVentWithFltMod_read)
  HmiDefrstMaxReqForClima_HmiDefrstMaxReqForClima/1 -> HvacCtrlrAirMFlowFrnt_Runnable_sys/87(HmiDefrstMaxReqForClima_HmiDefrstMaxReqForClima_read)
  HmiHvacFanLvlFrntForClima_HmiHvacFanLvlFrntForClima/1 -> HvacCtrlrAirMFlowFrnt_Runnable_sys/88(HmiHvacFanLvlFrntForClima_HmiHvacFanLvlFrntForClima_read)
  HmiHvacFanLvlFrntForClima_HmiHvacFanLvlFrntRiForClima/1 -> HvacCtrlrAirMFlowFrnt_Runnable_sys/89(HmiHvacFanLvlFrntForClima_HmiHvacFanLvlFrntRiForClima_read)
  HmiHvacFanLvlReForClima_HmiHvacFanLvlReForClima/1 -> HvacCtrlrAirMFlowFrnt_Runnable_sys/90(HmiHvacFanLvlReForClima_HmiHvacFanLvlReForClima_read)
  HmiHvacFanLvlReForClima_HmiHvacFanLvlReRiForClima/1 -> HvacCtrlrAirMFlowFrnt_Runnable_sys/91(HmiHvacFanLvlReForClima_HmiHvacFanLvlReRiForClima_read)
  HmiMaxACReqForClima_HmiMaxACReqForClima/1 -> HvacCtrlrAirMFlowFrnt_Runnable_sys/92(HmiMaxACReqForClima_HmiMaxACReqForClima_read)
  HvacAirMFlowEstimdForCtrlr_HvacAirMFlowEstimdForCtrlr/1 -> HvacCtrlrAirMFlowFrnt_Runnable_sys/93(HvacAirMFlowEstimdForCtrlr_HvacAirMFlowEstimdForCtrlr_read)
  HvacAirMFlowEstimdForCtrlrRe_HvacAirMFlowEstimdForCtrlrRe/1 -> HvacCtrlrAirMFlowFrnt_Runnable_sys/94(HvacAirMFlowEstimdForCtrlrRe_HvacAirMFlowEstimdForCtrlrRe_read)
  HvacAirMFlowMaxEstimdRe_HvacAirMFlowMaxEstimdRe/1 -> HvacCtrlrAirMFlowFrnt_Runnable_sys/95(HvacAirMFlowMaxEstimdRe_HvacAirMFlowMaxEstimdRe_read)
  HvacAirMFlowReqMaxAvl_HvacAirMFlowReqMaxAvl/1 -> HvacCtrlrAirMFlowFrnt_Runnable_sys/96(HvacAirMFlowReqMaxAvl_HvacAirMFlowReqMaxAvl_read)
  ManModeForRowFirstLeForModelDial_ManModeForRowFirstLeForModelDial/1 -> HvacCtrlrAirMFlowFrnt_Runnable_sys/97(ManModeForRowFirstLeForModelDial_ManModeForRowFirstLeForModelDial_read)
  ManModeForRowThrdForModelDial_ManModeForRowThrdForModelDial/1 -> HvacCtrlrAirMFlowFrnt_Runnable_sys/98(ManModeForRowThrdForModelDial_ManModeForRowThrdForModelDial_read)
  PrkgClimaOnReq_PrkgClimaOnReq/1 -> HvacCtrlrAirMFlowFrnt_Runnable_sys/99(PrkgClimaOnReq_PrkgClimaOnReq_read)
  PrkgClimaOnReqRe_PrkgClimaOnReqRe/1 -> HvacCtrlrAirMFlowFrnt_Runnable_sys/100(PrkgClimaOnReqRe_PrkgClimaOnReqRe_read)
  HvacCtrlrAirMFlowFrnt_Runnable_sys/1(HvacFlowReReqForRowThrdAtFlr_HvacFlowReReqForRowThrdAtFlr_write) -> HvacFlowReReqForRowThrdAtFlr_HvacFlowReReqForRowThrdAtFlr/1
  HvacCtrlrAirMFlowFrnt_Runnable_sys/2(HvacFlowReReqForRowThrdAtVent_HvacFlowReReqForRowThrdAtVent_write) -> HvacFlowReReqForRowThrdAtVent_HvacFlowReReqForRowThrdAtVent/1
  HvacCtrlrAirMFlowFrnt_Runnable_sys/3(HvacTarFlowTotRe_HvacTarFlowTotRe_write) -> HvacTarFlowTotRe_HvacTarFlowTotRe/1
  HvacCtrlrAirMFlowFrnt_Runnable_sys/4(HvacActFlowTot_HvacActFlowTot_write) -> HvacActFlowTot_HvacActFlowTot/1
  HvacCtrlrAirMFlowFrnt_Runnable_sys/5(HvacCtrlrFanBoostReqLe_HvacCtrlrFanBoostReqLe_write) -> HvacCtrlrFanBoostReqLe_HvacCtrlrFanBoostReqLe/1
  HvacCtrlrAirMFlowFrnt_Runnable_sys/6(HvacCtrlrFanBoostReqRi_HvacCtrlrFanBoostReqRi_write) -> HvacCtrlrFanBoostReqRi_HvacCtrlrFanBoostReqRi/1
  HvacCtrlrAirMFlowFrnt_Runnable_sys/7(HvacCtrlrFanReqFrnt_HvacCtrlrFanReqFrnt_write) -> HvacCtrlrFanReqFrnt_HvacCtrlrFanReqFrnt/1
  HvacCtrlrAirMFlowFrnt_Runnable_sys/8(HvacCtrlrReqForRowFirstForActrOsaForFanLvlLo_HvacCtrlrReqForRowFirstForActrOsaForFanLvlLo_write) -> HvacCtrlrReqForRowFirstForActrOsaForFanLvlLo_HvacCtrlrReqForRowFirstForActrOsaForFanLvlLo/1
  HvacCtrlrAirMFlowFrnt_Runnable_sys/9(HvacCtrlrReqForRowFirstForActrRecircForFanLvlLo_HvacCtrlrReqForRowFirstForActrRecircForFanLvlLo_write) -> HvacCtrlrReqForRowFirstForActrRecircForFanLvlLo_HvacCtrlrReqForRowFirstForActrRecircForFanLvlLo/1
  HvacCtrlrAirMFlowFrnt_Runnable_sys/10(HvacCtrlrReqFrntForOsaAndRecircForFanLvlLo_HvacCtrlrReqFrntForOsaAndRecircForFanLvlLo_write) -> HvacCtrlrReqFrntForOsaAndRecircForFanLvlLo_HvacCtrlrReqFrntForOsaAndRecircForFanLvlLo/1
  HvacCtrlrAirMFlowFrnt_Runnable_sys/11(HvacFanLvlLoFlg_HvacFanLvlLoFlg_write) -> HvacFanLvlLoFlg_HvacFanLvlLoFlg/1
  HvacCtrlrAirMFlowFrnt_Runnable_sys/12(HvacFlowFrntRednEnad_HvacFlowFrntRednEnad_write) -> HvacFlowFrntRednEnad_HvacFlowFrntRednEnad/1
  HvacCtrlrAirMFlowFrnt_Runnable_sys/13(HvacTarFlowTot_HvacTarFlowTot_write) -> HvacTarFlowTot_HvacTarFlowTot/1
  HvacCtrlrAirMFlowFrnt_Runnable_sys/14(UBoostReqByClima_Ngcc_UBoostReqByClima_write) -> UBoostReqByClima_Ngcc_UBoostReqByClima/1
  HvacCtrlrAirMFlowFrnt_Runnable/1 -> HvacCtrlrAirMFlowFrnt_Runnable_sys/Trigger

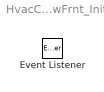
== system HvacCtrlrAirMFlowFrnt/HvacCtrlrAirMFlowFrnt_Init ==
  Event Listener : EventListener  EventName=reset
